simulink model "controller_observer_AllAgents_v5"  (Simulink 8.4) (continued)
== system controller_observer_AllAgents_v5/Controller == (continued)
  CornerClass1Pod2_Cooling_Mux/1 -> CornerClass1Pod2_Cooling/1(In1)
  [On_Off] -> CornerClass2Pod1_Cooling/Enable
  [On_Off] -> CornerClass1Pod2_Cooling/Enable
  [On_Off] -> CornerClass2Pod2_Cooling/Enable
  [On_Off] -> CornerClass1Pod3_Cooling/Enable
  [On_Off] -> CornerClass2Pod3_Cooling/Enable
  [On_Off] -> MultiClass1Pod1_Cooling/Enable
  [On_Off] -> MultiClass2Pod1_Cooling/Enable
  [On_Off] -> MultiClass1Pod2_Cooling/Enable
  [On_Off] -> MultiClass2Pod2_Cooling/Enable
  [On_Off] -> MultiClass1Pod3_Cooling/Enable
  [On_Off] -> MultiClass2Pod3_Cooling/Enable
  [On_Off] -> Mech_Cooling/Enable
  [On_Off] -> MainCorridor_Cooling/Enable
  [On_Off] -> CorridorPod3_Cooling/Enable
  [On_Off] -> CorridorPod2_Cooling/Enable
  [On_Off] -> CorridorPod1_Cooling/Enable
  [On_Off] -> ComputerClass_Cooling/Enable
  [On_Off] -> LibraryMediaCenter_Cooling/Enable
  [On_Off] -> Offices_Cooling/Enable
  [On_Off] -> Lobby_Cooling/Enable
  [On_Off] -> Bath_Cooling/Enable
  [On_Off] -> Gym_Cooling/Enable
  [On_Off] -> Kitchen_Cooling/Enable
  [On_Off] -> Cafeteria_Cooling/Enable
  [On_Off] -> CornerClass2Pod1_Heating/Enable
  [On_Off] -> CornerClass1Pod2_Heating/Enable
  [On_Off] -> CornerClass2Pod2_Heating/Enable
  [On_Off] -> CornerClass1Pod3_Heating/Enable
  [On_Off] -> CornerClass2Pod3_Heating/Enable
  [On_Off] -> MultiClass2Pod3_Heating/Enable
  [On_Off] -> MultiClass1Pod3_Heating/Enable
  [On_Off] -> MultiClass2Pod2_Heating/Enable
  [On_Off] -> MultiClass1Pod2_Heating/Enable
  [On_Off] -> MultiClass2Pod1_Heating/Enable
  [On_Off] -> MultiClass1Pod1_Heating/Enable
  [On_Off] -> CorridorPod1_Heating/Enable
  [On_Off] -> CorridorPod2_Heating/Enable
  [On_Off] -> CorridorPod3_Heating/Enable
  [On_Off] -> MainCorridor_Heating/Enable
  [On_Off] -> Mech_Heating/Enable
  [On_Off] -> Lobby_Heating/Enable
  [On_Off] -> Offices_Heating/Enable
  [On_Off] -> LibraryMediaCenter_Heating/Enable
  [On_Off] -> ComputerClass_Heating/Enable
  [On_Off] -> Cafeteria_Heating/Enable
  [On_Off] -> Kitchen_Heating/Enable
  [On_Off] -> Gym_Heating/Enable
  [On_Off] -> Bath_Heating/Enable
  Fault_Tz_CornerClass1Pod1/1(Out) -> [TzCornerClass1Pod1]
  Fault_Tsa_CornerClass1Pod1/1(Out) -> [TsaCornerClass1Pod1]
  Fault_Tc_CornerClass1Pod1_Cooling/1(Out) -> [TcCornerClass1Pod1_Cooling]
  Fault_Tc_CornerClass1Pod1_Heating/1(Out) -> [TcCornerClass1Pod1_Heating]
  Fault_Tz_CornerClass2Pod1/1(Out) -> [TzCornerClass2Pod1]
  Fault_Tsa_CornerClass2Pod1/1(Out) -> [TsaCornerClass2Pod1]
  Fault_Tc_CornerClass2Pod1_Cooling/1(Out) -> [TcCornerClass2Pod1_Cooling]
  Fault_Tc_CornerClass2Pod1_Heating/1(Out) -> [TcCornerClass2Pod1_Heating]
  Fault_Tz_CornerClass1Pod2/1(Out) -> [TzCornerClass1Pod2]
  Fault_Tsa_CornerClass1Pod2/1(Out) -> [TsaCornerClass1Pod2]
  Fault_Tc_CornerClass1Pod2_Cooling/1(Out) -> [TcCornerClass1Pod2_Cooling]
  Fault_Tc_CornerClass1Pod2_Heating/1(Out) -> [TcCornerClass1Pod2_Heating]
  Fault_Tz_CornerClass2Pod2/1(Out) -> [TzCornerClass2Pod2]
  Fault_Tsa_CornerClass2Pod2/1(Out) -> [TsaCornerClass2Pod2]
  Fault_Tc_CornerClass2Pod2_Cooling/1(Out) -> [TcCornerClass2Pod2_Cooling]
  Fault_Tc_CornerClass2Pod2_Heating/1(Out) -> [TcCornerClass2Pod2_Heating]
  Fault_Tz_CornerClass1Pod3/1(Out) -> [TzCornerClass1Pod3]
  Fault_Tsa_CornerClass1Pod3/1(Out) -> [TsaCornerClass1Pod3]
  Fault_Tc_CornerClass1Pod3_Cooling/1(Out) -> [TcCornerClass1Pod3_Cooling]
  Fault_Tc_CornerClass1Pod3_Heating/1(Out) -> [TcCornerClass1Pod3_Heating]
  Fault_Tz_CornerClass2Pod3/1(Out) -> [TzCornerClass2Pod3]
  Fault_Tsa_CornerClass2Pod3/1(Out) -> [TsaCornerClass2Pod3]
  Fault_Tc_CornerClass2Pod3_Cooling/1(Out) -> [TcCornerClass2Pod3_Cooling]
  Fault_Tc_CornerClass2Pod3_Heating/1(Out) -> [TcCornerClass2Pod3_Heating]
  Fault_Tz_MultiClass1Pod1/1(Out) -> [TzMultiClass1Pod1]
  Fault_Tsa_MultiClass1Pod1/1(Out) -> [TsaMultiClass1Pod1]
  Fault_Tc_MultiClass1Pod1_Cooling/1(Out) -> [TcMultiClass1Pod1_Cooling]
  Fault_Tc_MultiClass1Pod1_Heating/1(Out) -> [TcMultiClass1Pod1_Heating]
  Fault_Tz_MultiClass2Pod1/1(Out) -> [TzMultiClass2Pod1]
  Fault_Tsa_MultiClass2Pod1/1(Out) -> [TsaMultiClass2Pod1]
  Fault_Tc_MultiClass2Pod1_Cooling/1(Out) -> [TcMultiClass2Pod1_Cooling]
  Fault_Tc_MultiClass2Pod1_Heating/1(Out) -> [TcMultiClass2Pod1_Heating]
  Fault_Tz_MultiClass1Pod2/1(Out) -> [TzMultiClass1Pod2]
  Fault_Tsa_MultiClass1Pod2/1(Out) -> [TsaMultiClass1Pod2]
  Fault_Tc_MultiClass1Pod2_Cooling/1(Out) -> [TcMultiClass1Pod2_Cooling]
  Fault_Tc_MultiClass1Pod2_Heating/1(Out) -> [TcMultiClass1Pod2_Heating]
  Fault_Tc_MultiClass2Pod2_Cooling/1(Out) -> [TcMultiClass2Pod2_Cooling]
  Fault_Tsa_MultiClass2Pod2/1(Out) -> [TsaMultiClass2Pod2]
  Fault_Tz_MultiClass2Pod2/1(Out) -> [TzMultiClass2Pod2]
  Fault_Tc_MultiClass2Pod2_Heating/1(Out) -> [TcMultiClass2Pod2_Heating]
  Fault_Tz_MultiClass1Pod3/1(Out) -> [TzMultiClass1Pod3]
  Fault_Tc_MultiClass1Pod3_Cooling/1(Out) -> [TcMultiClass1Pod3_Cooling]
  Fault_Tsa_MultiClass1Pod3/1(Out) -> [TsaMultiClass1Pod3]
  Fault_Tc_MultiClass1Pod3_Heating/1(Out) -> [TcMultiClass1Pod3_Heating]
  Fault_Tz_MultiClass2Pod3/1(Out) -> [TzMultiClass2Pod3]
  Fault_Tc_MultiClass2Pod3_Cooling/1(Out) -> [TcMultiClass2Pod3_Cooling]
  Fault_Tsa_MultiClass2Pod3/1(Out) -> [TsaMultiClass2Pod3]
  Fault_Tc_MultiClass2Pod3_Heating/1(Out) -> [TcMultiClass2Pod3_Heating]
  Fault_Tz_CorridorPod1/1(Out) -> [TzCorridorPod1]
  Fault_Tsa_CorridorPod1/1(Out) -> [TsaCorridorPod1]
  Fault_Tc_CorridorPod1_Heating/1(Out) -> [TcCorridorPod1_Heating]
  Fault_Tc_CorridorPod1_Cooling/1(Out) -> [TcCorridorPod1_Cooling]
  Fault_Tz_CorridorPod2/1(Out) -> [TzCorridorPod2]
  Fault_Tc_CorridorPod2_Heating/1(Out) -> [TcCorridorPod2_Heating]
  Fault_Tc_CorridorPod2_Cooling/1(Out) -> [TcCorridorPod2_Cooling]
  Fault_Tsa_CorridorPod2/1(Out) -> [TsaCorridorPod2]
  Fault_Tz_CorridorPod3/1(Out) -> [TzCorridorPod3]
  Fault_Tc_CorridorPod3_Cooling/1(Out) -> [TcCorridorPod3_Cooling]
  Fault_Tsa_CorridorPod3/1(Out) -> [TsaCorridorPod3]
  Fault_Tc_CorridorPod3_Heating/1(Out) -> [TcCorridorPod3_Heating]
  Fault_Tc_MainCorridor_Heating/1(Out) -> [TcMainCorridor_Heating]
  Fault_Tc_MainCorridor_Cooling/1(Out) -> [TcMainCorridor_Cooling]
  Fault_Tz_MainCorridor/1(Out) -> [TzMainCorridor]
  Fault_Tsa_MainCorridor/1(Out) -> [TsaMainCorridor]
  Fault_Tz_Mech/1(Out) -> [TzMech]
  Fault_Tc_Mech_Cooling/1(Out) -> [TcMech_Cooling]
  Fault_Tc_Mech_Heating/1(Out) -> [TcMech_Heating]
  Fault_Tsa_Mech/1(Out) -> [TsaMech]
  Fault_Tc_ComputerClass_Heating/1(Out) -> [TcComputerClass_Heating]
  Fault_Tsa_ComputerClass/1(Out) -> [TsaComputerClass]
  Fault_Tz_ComputerClass/1(Out) -> [TzComputerClass]
  Fault_Tc_ComputerClass_Cooling/1(Out) -> [TcComputerClass_Cooling]
  Fault_Tc_LibraryMediaCenter_Cooling/1(Out) -> [TcLibrary_Cooling]
  Fault_Tsa_LibraryMediaCenter/1(Out) -> [TsaLibrary]
  Fault_Tz_LibraryMediaCenter/1(Out) -> [TzLibrary]
  Fault_Tc_LibraryMediaCenter_Heating/1(Out) -> [TcLibrary_Heating]
  Fault_Tc_Offices_Heating/1(Out) -> [TcOffices_Heating]
  Fault_Tz_Offices/1(Out) -> [TzOffices]
  Fault_Tsa_Offices/1(Out) -> [TsaOffices]
  Fault_Tc_Offices_Cooling/1(Out) -> [TcOffices_Cooling]
  Fault_Tc_Lobby_Cooling/1(Out) -> [TcLobby_Cooling]
  Fault_Tc_Lobby_Heating/1(Out) -> [TcLobby_Heating]
  Fault_Tsa_Lobby/1(Out) -> [TsaLobby]
  Fault_Tz_Lobby/1(Out) -> [TzLobby]
  Fault_Tc_Cafeteria_Cooling/1(Out) -> [TcCafeteria_Cooling]
  Fault_Tc_Cafeteria_Heating/1(Out) -> [TcCafeteria_Heating]
  Fault_Tz_Cafeteria/1(Out) -> [TzCafeteria]
  Fault_Tsa_Cafeteria/1(Out) -> [TsaCafeteria]
  Fault_Tc_Kitchen_Cooling/1(Out) -> [TcKitchen_Cooling]
  Fault_Tc_Kitchen_Heating/1(Out) -> [TcKitchen_Heating]
  Fault_Tsa_Kitchen/1(Out) -> [TsaKitchen]
  Fault_Tz_Kitchen/1(Out) -> [TzKitchen]
  Fault_Tsa_Gym/1(Out) -> [TsaGym]
  Fault_Tc_Gym_Cooling/1(Out) -> [TcGym_Cooling]
  Fault_Tz_Gym/1(Out) -> [TzGym]
  Fault_Tc_Gym_Heating/1(Out) -> [TcGym_Heating]
  Fault_Tz_Bath/1(Out) -> [TzBath]
  Fault_Tsa_Bath/1(Out) -> [TsaBath]
  Fault_Tc_Bath_Cooling/1(Out) -> [TcBath_Cooling]
  Fault_Tc_Bath_Heating/1(Out) -> [TcBath_Heating]
  Fault_Tst_Cooling/1(Out) -> [Tst_Cooling]
  Fault_Tamb/1(Out) -> [Tamb]
  Fault_Tst_Heating/1(Out) -> [Tst_Heating]
  Demux/104 -> [On_Off]
  Fault_mc_CornerClass1Pod1_Cooling/1(Out) -> Mux2/1
  Fault_mc_CornerClass2Pod1_Cooling/1(Out) -> Mux2/2
  Fault_mc_CornerClass1Pod2_Cooling/1(Out) -> Mux2/3
  Fault_mc_CornerClass2Pod2_Cooling/1(Out) -> Mux2/4
  Fault_mc_CornerClass1Pod3_Cooling/1(Out) -> Mux2/5
  Fault_mc_CornerClass2Pod3_Cooling/1(Out) -> Mux2/6
  Fault_mc_MultiClass1Pod2_Cooling/1(Out) -> Mux2/9
  Fault_mc_MultiClass1Pod1_Cooling/1(Out) -> Mux2/7
  Fault_mc_MultiClass1Pod3_Cooling/1(Out) -> Mux2/11
  Fault_mc_MultiClass2Pod1_Cooling/1(Out) -> Mux2/8
  Fault_mc_MultiClass2Pod3_Cooling/1(Out) -> Mux2/12
  Fault_mc_MultiClass2Pod2_Cooling/1(Out) -> Mux2/10
  Fault_mc_CorridorPod1_Cooling/1(Out) -> Mux2/13
  Fault_mc_CorridorPod3_Cooling/1(Out) -> Mux2/15
  Fault_mc_Mech_Cooling/1(Out) -> Mux2/17
  Fault_mc_CorridorPod2_Cooling/1(Out) -> Mux2/14
  Fault_mc_ComputerClass_Cooling/1(Out) -> Mux2/18
  Fault_mc_MainCorridor_Cooling/1(Out) -> Mux2/16
  Fault_mc_Gym_Cooling/1(Out) -> Mux2/24
  Fault_mc_Kitchen_Cooling/1(Out) -> Mux2/23
  Fault_mc_Lobby_Cooling/1(Out) -> Mux2/21
  Fault_mc_Cafeteria_Cooling/1(Out) -> Mux2/22
  Fault_mc_LibraryMediaCenter_Cooling/1(Out) -> Mux2/19
  Fault_mc_Offices_Cooling/1(Out) -> Mux2/20
  Fault_mc_Bath_Cooling/1(Out) -> Mux2/25
  Fault_mc_Bath_Heating/1(Out) -> Mux2/50
  Fault_mc_ComputerClass_Heating/1(Out) -> Mux2/43
  Fault_mc_CornerClass2Pod2_Heating/1(Out) -> Mux2/29
  Fault_mc_CorridorPod1_Heating/1(Out) -> Mux2/38
  Fault_mc_CornerClass1Pod3_Heating/1(Out) -> Mux2/30
  Fault_mc_CornerClass2Pod1_Heating/1(Out) -> Mux2/27
  Fault_mc_CornerClass1Pod1_Heating/1(Out) -> Mux2/26
  Fault_mc_CornerClass1Pod2_Heating/1(Out) -> Mux2/28
  Fault_mc_Cafeteria_Heating/1(Out) -> Mux2/47
  Fault_mc_CornerClass2Pod3_Heating/1(Out) -> Mux2/31
  Fault_mc_MainCorridor_Heating/1(Out) -> Mux2/41
  Fault_mc_Kitchen_Heating/1(Out) -> Mux2/48
  Fault_mc_Gym_Heating/1(Out) -> Mux2/49
  Fault_mc_Lobby_Heating/1(Out) -> Mux2/46
  Fault_mc_CorridorPod3_Heating/1(Out) -> Mux2/40
  Fault_mc_CorridorPod2_Heating/1(Out) -> Mux2/39
  Fault_mc_LibraryMediaCenter_Heating/1(Out) -> Mux2/44
  Fault_mc_Mech_Heating/1(Out) -> Mux2/42
  Fault_mc_MultiClass1Pod1_Heating/1(Out) -> Mux2/32
  Fault_mc_MultiClass2Pod2_Heating/1(Out) -> Mux2/35
  Fault_mc_MultiClass1Pod3_Heating/1(Out) -> Mux2/36
  Fault_mc_MultiClass2Pod3_Heating/1(Out) -> Mux2/37
  Fault_mc_MultiClass1Pod2_Heating/1(Out) -> Mux2/34
  Fault_mc_MultiClass2Pod1_Heating/1(Out) -> Mux2/33
  Fault_mc_Offices_Heating/1(Out) -> Mux2/45
  CorridorPod2_Mux1/1 -> CorridorPod2_Agent/1(In1)
  [Tamb] -> MainCorridor_Mux1/2
  [TcLibrary_Cooling] -> LibraryMediaCenter_Mux1/3
  LibraryMediaCenter_Mux1/1 -> LibraryMediaCenter_Agent/1(In1)
  [TzMainCorridor] -> MainCorridor_Mux1/1
  MainCorridor_Mux1/1 -> MainCorridor_Agent/1(In1)
  [Tamb] -> LibraryMediaCenter_Mux1/2
  [Tamb] -> CorridorPod3_Mux1/2
  [TzMultiClass2Pod3] -> MultiClass2Pod3_Mux1/1
  MultiClass1Pod2_Mux1/1 -> MultiClass1Pod2_Agent/1(In1)
  [Tamb] -> MultiClass1Pod2_Mux1/2
  [TzMultiClass2Pod1] -> MultiClass2Pod1_Mux1/1
  [Tamb] -> MultiClass2Pod1_Mux1/2
  [TcCornerClass2Pod3_Cooling] -> CornerClass2Pod3_Mux1/3
  [Tamb] -> MultiClass1Pod1_Mux1/2
  [TcCornerClass1Pod3_Cooling] -> CornerClass1Pod3_Mux1/3
  [Tamb] -> CornerClass2Pod2_Mux1/2
  [Tamb] -> CornerClass1Pod2_Mux1/2
  Cafeteria_Mux1/1 -> Cafeteria_Agent/1(In1)
  [Tamb] -> MultiClass2Pod2_Mux1/2
  CornerClass1Pod3_Mux1/1 -> CornerClass1Pod3_Agent/1(In1)
  [Tamb] -> MultiClass2Pod3_Mux1/2
  [Tamb] -> Bath_Mux1/2
  [TcMultiClass1Pod1_Cooling] -> MultiClass1Pod1_Mux1/3
  Lobby_Mux1/1 -> Lobby_Agent/1(In1)
  [Tamb] -> Lobby_Mux1/2
  [TcCafeteria_Cooling] -> Cafeteria_Mux1/3
  Offices_Mux1/1 -> Offices_Agent/1(In1)
  [TcMech_Cooling] -> Mech_Mux1/3
  [Tamb] -> Cafeteria_Mux1/2
  [TzMultiClass1Pod1] -> MultiClass1Pod1_Mux1/1
  [TcComputerClass_Cooling] -> ComputerClass_Mux1/3
  [Tamb] -> CornerClass1Pod3_Mux1/2
  [Tamb] -> CorridorPod1_Mux1/2
  [TzOffices] -> Offices_Mux1/1
  [Tamb] -> Gym_Mux1/2
  [TzMech] -> Mech_Mux1/1
  ComputerClass_Mux1/1 -> ComputerClass_Agent/1(In1)
  [TzCorridorPod1] -> CorridorPod1_Mux1/1
  [Tamb] -> ComputerClass_Mux1/2
  MultiClass1Pod1_Mux1/1 -> MultiClass1Pod1_Agent/1(In1)
  Bath_Mux1/1 -> Bath_Agent/1(In1)
  MultiClass2Pod3_Mux1/1 -> MultiClass2Pod3_Agent/1(In1)
  [TzLibrary] -> LibraryMediaCenter_Mux1/1
  [TzLobby] -> Lobby_Mux1/1
  [Tst_Cooling] -> CornerClass1Pod1_Mux1/6
  [Tamb] -> MultiClass1Pod3_Mux1/2
  [TzCornerClass1Pod2] -> CornerClass1Pod2_Mux1/1
  [TzCorridorPod3] -> CorridorPod3_Mux1/1
  [TcCorridorPod2_Cooling] -> CorridorPod2_Mux1/3
  MultiClass2Pod2_Mux1/1 -> MultiClass2Pod2_Agent/1(In1)
  CornerClass1Pod1_Mux1/1 -> CornerClass1Pod1_Agent/1(In1)
  [TzNeighborCornerClass1Pod1] -> CornerClass1Pod1_Mux1/8
  [TcLobby_Cooling] -> Lobby_Mux1/3
  [TzGym] -> Gym_Mux1/1
  [TcMultiClass2Pod2_Cooling] -> MultiClass2Pod2_Mux1/3
  [TzCornerClass1Pod3] -> CornerClass1Pod3_Mux1/1
  MultiClass2Pod1_Mux1/1 -> MultiClass2Pod1_Agent/1(In1)
  [TcGym_Cooling] -> Gym_Mux1/3
  [TzMultiClass1Pod3] -> MultiClass1Pod3_Mux1/1
  [TcCornerClass2Pod2_Cooling] -> CornerClass2Pod2_Mux1/3
  [Tamb] -> CornerClass1Pod1_Mux1/2
  CorridorPod1_Mux1/1 -> CorridorPod1_Agent/1(In1)
  [TzMultiClass1Pod2] -> MultiClass1Pod2_Mux1/1
  Kitchen_Mux1/1 -> Kitchen_Agent/1(In1)
  [Tamb] -> CornerClass2Pod1_Mux1/2
  Gym_Mux1/1 -> Gym_Agent/1(In1)
  [TsaCornerClass1Pod1] -> CornerClass1Pod1_Mux1/5
  [TzCornerClass2Pod3] -> CornerClass2Pod3_Mux1/1
  [TcCornerClass1Pod1_Cooling] -> CornerClass1Pod1_Mux1/3
  CornerClass2Pod3_Mux1/1 -> CornerClass2Pod3_Agent/1(In1)
  [TcKitchen_Cooling] -> Kitchen_Mux1/3
  [TzCornerClass2Pod1] -> CornerClass2Pod1_Mux1/1
  [Tamb] -> CorridorPod2_Mux1/2
  [TcMultiClass1Pod3_Cooling] -> MultiClass1Pod3_Mux1/3
  CornerClass2Pod2_Mux1/1 -> CornerClass2Pod2_Agent/1(In1)
  CornerClass1Pod2_Mux1/1 -> CornerClass1Pod2_Agent/1(In1)
  [Tamb] -> Mech_Mux1/2
  [Tamb] -> Kitchen_Mux1/2
  [TcMultiClass2Pod1_Cooling] -> MultiClass2Pod1_Mux1/3
  CorridorPod3_Mux1/1 -> CorridorPod3_Agent/1(In1)
  [Tamb] -> Offices_Mux1/2
  [Tamb] -> CornerClass2Pod3_Mux1/2
  [TzMultiClass2Pod2] -> MultiClass2Pod2_Mux1/1
  [TzKitchen] -> Kitchen_Mux1/1
  [TzCafeteria] -> Cafeteria_Mux1/1
  [TcOffices_Cooling] -> Offices_Mux1/3
  [TcCorridorPod1_Cooling] -> CorridorPod1_Mux1/3
  [TcMultiClass1Pod2_Cooling] -> MultiClass1Pod2_Mux1/3
  [TcCornerClass2Pod1_Cooling] -> CornerClass2Pod1_Mux1/3
  [TcMultiClass2Pod3_Cooling] -> MultiClass2Pod3_Mux1/3
  [TcCornerClass1Pod2_Cooling] -> CornerClass1Pod2_Mux1/3
  [TzBath] -> Bath_Mux1/1
  MultiClass1Pod3_Mux1/1 -> MultiClass1Pod3_Agent/1(In1)
  Mech_Mux1/1 -> Mech_Agent/1(In1)
  [TzCorridorPod2] -> CorridorPod2_Mux1/1
  [TzCornerClass2Pod2] -> CornerClass2Pod2_Mux1/1
  [TzCornerClass1Pod1] -> CornerClass1Pod1_Mux1/1
  [TcBath_Cooling] -> Bath_Mux1/3
  CornerClass2Pod1_Mux1/1 -> CornerClass2Pod1_Agent/1(In1)
  [TcCorridorPod3_Cooling] -> CorridorPod3_Mux1/3
  [TzComputerClass] -> ComputerClass_Mux1/1
  [TcMainCorridor_Cooling] -> MainCorridor_Mux1/3
  [On_Off] -> CornerClass2Pod1_Agent/Enable
  [On_Off] -> CornerClass1Pod2_Agent/Enable
  [On_Off] -> CornerClass2Pod2_Agent/Enable
  [On_Off] -> CornerClass1Pod3_Agent/Enable
  [On_Off] -> CornerClass2Pod3_Agent/Enable
  [On_Off] -> MultiClass1Pod1_Agent/Enable
  [On_Off] -> MultiClass2Pod1_Agent/Enable
  [On_Off] -> MultiClass1Pod2_Agent/Enable
  [On_Off] -> MultiClass2Pod2_Agent/Enable
  [On_Off] -> MultiClass1Pod3_Agent/Enable
  [On_Off] -> MultiClass2Pod3_Agent/Enable
  [On_Off] -> CorridorPod1_Agent/Enable
  [On_Off] -> CorridorPod2_Agent/Enable
  [On_Off] -> CorridorPod3_Agent/Enable
  [On_Off] -> MainCorridor_Agent/Enable
  [On_Off] -> Mech_Agent/Enable
  [On_Off] -> ComputerClass_Agent/Enable
  [On_Off] -> LibraryMediaCenter_Agent/Enable
  [On_Off] -> Offices_Agent/Enable
  [On_Off] -> Lobby_Agent/Enable
  [On_Off] -> Cafeteria_Agent/Enable
  [On_Off] -> Kitchen_Agent/Enable
  [On_Off] -> Gym_Agent/Enable
  [On_Off] -> Bath_Agent/Enable
  Mux3/1 -> [DzNeighborCornerClass1Pod1]
  [DzMultiClass1Pod1] -> Mux3/1
  [DzCorridorPod1] -> Mux3/2
  Mux4/1 -> [DzNeighborCornerClass2Pod1]
  Mux5/1 -> [DzNeighborCornerClass1Pod2]
  [DzMultiClass1Pod2] -> Mux5/1
  [DzCorridorPod2] -> Mux5/2
  Mux6/1 -> [DzNeighborCornerClass2Pod2]
  Mux7/1 -> [DzNeighborCornerClass1Pod3]
  [DzMultiClass1Pod3] -> Mux7/1
  [DzCorridorPod3] -> Mux7/2
  Mux8/1 -> [DzNeighborCornerClass2Pod3]
  [DzCorridorPod1] -> Mux4/1
  [DzMultiClass2Pod1] -> Mux4/2
  [DzCorridorPod2] -> Mux6/1
  [DzMultiClass2Pod2] -> Mux6/2
  [DzCorridorPod3] -> Mux8/1
  [DzMultiClass2Pod3] -> Mux8/2
  Mux9/1 -> [DzNeighborMultiClass1Pod1]
  Mux10/1 -> [DzNeighborMultiClass2Pod1]
  Mux11/1 -> [DzNeighborMultiClass1Pod2]
  Mux12/1 -> [DzNeighborMultiClass2Pod2]
  Mux13/1 -> [DzNeighborMultiClass1Pod3]
  [DzCorridorPod3] -> Mux13/2
  Mux14/1 -> [DzNeighborMultiClass2Pod3]
  [DzCornerClass1Pod1] -> Mux9/3
  [DzCorridorPod1] -> Mux9/2
  [DzLobby] -> Mux9/1
  [DzCorridorPod1] -> Mux10/1
  [DzMainCorridor] -> Mux10/2
  [DzCornerClass2Pod1] -> Mux10/3
  [DzCorridorPod2] -> Mux11/2
  [DzCornerClass1Pod2] -> Mux11/3
  [DzMainCorridor] -> Mux11/1
  [DzCorridorPod2] -> Mux12/1
  [DzMainCorridor] -> Mux12/2
  [DzCornerClass2Pod2] -> Mux12/3
  [DzLibrary] -> Mux13/1
  [DzCornerClass1Pod3] -> Mux13/3
  [DzCorridorPod3] -> Mux14/1
  [DzComputerClass] -> Mux14/2
  [DzCornerClass2Pod3] -> Mux14/3
  Mux15/1 -> [DzNeighborCorridorPod1]
  [DzCornerClass1Pod1] -> Mux15/1
  [DzMultiClass2Pod1] -> Mux15/4
  Mux16/1 -> [DzNeighborCorridorPod2]
  [DzCornerClass1Pod2] -> Mux16/1
  [DzMultiClass2Pod2] -> Mux16/4
  Mux17/1 -> [DzNeighborCorridorPod3]
  [DzCornerClass1Pod3] -> Mux17/1
  Mux18/1 -> [DzNeighborMainCorridor]
  Mux19/1 -> [DzNeighborMech]
  [DzMainCorridor] -> Mux15/3
  [DzCornerClass2Pod1] -> Mux15/5
  [DzMultiClass1Pod1] -> Mux15/2
  [DzMultiClass1Pod2] -> Mux16/2
  [DzMainCorridor] -> Mux16/3
  [DzCornerClass2Pod2] -> Mux16/5
  [DzMultiClass1Pod3] -> Mux17/2
  [DzLibrary] -> Mux17/3
  [DzComputerClass] -> Mux17/4
  [DzMultiClass2Pod3] -> Mux17/5
  [DzCornerClass2Pod3] -> Mux17/6
  [DzBath] -> Mux18/3
  [DzMech] -> Mux18/2
  [DzLobby] -> Mux18/1
  [DzMultiClass2Pod2] -> Mux18/4
  [DzCorridorPod2] -> Mux18/5
  [DzMultiClass1Pod2] -> Mux18/6
  [DzCorridorPod1] -> Mux18/8
  [DzMultiClass2Pod1] -> Mux18/7
  [DzLobby] -> Mux19/1
  [DzOffices] -> Mux19/2
  [DzGym] -> Mux19/3
  [DzKitchen] -> Mux19/4
  [DzCafeteria] -> Mux19/5
  [DzMainCorridor] -> Mux19/7
  [DzBath] -> Mux19/6
  Mux20/1 -> [DzNeighborComputerClass]
  Mux21/1 -> [DzNeighborLibrary]
  [DzComputerClass] -> Mux21/2
  [DzCorridorPod3] -> Mux21/3
  Mux28/1 -> [DzNeighborOffices]
  Mux29/1 -> [DzNeighborLobby]
  [DzCorridorPod3] -> Mux20/1
  [DzLibrary] -> Mux20/2
  [DzMultiClass2Pod3] -> Mux20/3
  [DzMultiClass1Pod3] -> Mux21/4
  [DzBath] -> Mux21/1
  [DzLobby] -> Mux28/3
  [DzGym] -> Mux28/1
  [DzMech] -> Mux28/2
  [DzMainCorridor] -> Mux29/3
  [DzMultiClass1Pod1] -> Mux29/4
  [DzMech] -> Mux29/2
  [DzOffices] -> Mux29/1
  Mux30/1 -> [DzNeighborCafeteria]
  [DzKitchen] -> Mux30/1
  Mux31/1 -> [DzNeighborKitchen]
  [DzGym] -> Mux31/1
  Mux32/1 -> [DzNeighborGym]
  [DzOffices] -> Mux32/1
  Mux33/1 -> [DzNeighborBath]
  [DzMainCorridor] -> Mux33/1
  [DzMech] -> Mux33/2
  [DzLibrary] -> Mux33/4
  [DzCafeteria] -> Mux33/3
  [DzBath] -> Mux30/2
  [DzMech] -> Mux30/3
  [DzCafeteria] -> Mux31/2
  [DzMech] -> Mux31/3
  [DzKitchen] -> Mux32/2
  [DzMech] -> Mux32/3
  CornerClass1Pod1_Agent/1(Dz) -> [DzCornerClass1Pod1]
  CornerClass2Pod1_Agent/1(Dz) -> [DzCornerClass2Pod1]
  CornerClass1Pod2_Agent/1(Dz) -> [DzCornerClass1Pod2]
  CornerClass2Pod2_Agent/1(Dz) -> [DzCornerClass2Pod2]
  CornerClass1Pod3_Agent/1(Dz) -> [DzCornerClass1Pod3]
  CornerClass2Pod3_Agent/1(Dz) -> [DzCornerClass2Pod3]
  MultiClass1Pod1_Agent/1(Dz) -> [DzMultiClass1Pod1]
  MultiClass2Pod1_Agent/1(Dz) -> [DzMultiClass2Pod1]
  MultiClass1Pod3_Agent/1(Dz) -> [DzMultiClass1Pod3]
  MultiClass2Pod3_Agent/1(Dz) -> [DzMultiClass2Pod3]
  MultiClass1Pod2_Agent/1(Dz) -> [DzMultiClass1Pod2]
  MultiClass2Pod2_Agent/1(Dz) -> [DzMultiClass2Pod2]
  CorridorPod1_Agent/1(Dz) -> [DzCorridorPod1]
  CorridorPod2_Agent/1(Dz) -> [DzCorridorPod2]
  CorridorPod3_Agent/1(Dz) -> [DzCorridorPod3]
  MainCorridor_Agent/1(Dz) -> [DzMainCorridor]
  Mech_Agent/1(Dz) -> [DzMech]
  ComputerClass_Agent/1(Dz) -> [DzComputerClass]
  LibraryMediaCenter_Agent/1(Dz) -> [DzLibrary]
  Offices_Agent/1(Dz) -> [DzOffices]
  Lobby_Agent/1(Dz) -> [DzLobby]
  Cafeteria_Agent/1(Dz) -> [DzCafeteria]
  Kitchen_Agent/1(Dz) -> [DzKitchen]
  Gym_Agent/1(Dz) -> [DzGym]
  Bath_Agent/1(Dz) -> [DzBath]
  [TcCornerClass1Pod1_Heating] -> CornerClass1Pod1_Mux1/4
  [Tst_Heating] -> CornerClass1Pod1_Mux1/7
  [mcCornerClass1Pod1_Heating] -> CornerClass1Pod1_Mux1/10
  [mcCornerClass2Pod1_Cooling] -> CornerClass2Pod1_Mux1/9
  [TzNeighborCornerClass2Pod1] -> CornerClass2Pod1_Mux1/8
  [Tst_Heating] -> CornerClass2Pod1_Mux1/7
  [Tst_Cooling] -> CornerClass2Pod1_Mux1/6
  [TsaCornerClass2Pod1] -> CornerClass2Pod1_Mux1/5
  [TcCornerClass2Pod1_Heating] -> CornerClass2Pod1_Mux1/4
  [mcCornerClass2Pod1_Heating] -> CornerClass2Pod1_Mux1/10
  [mcCornerClass1Pod2_Cooling] -> CornerClass1Pod2_Mux1/9
  [TzNeighborCornerClass1Pod2] -> CornerClass1Pod2_Mux1/8
  [Tst_Cooling] -> CornerClass1Pod2_Mux1/6
  [TsaCornerClass1Pod2] -> CornerClass1Pod2_Mux1/5
  [TcCornerClass1Pod2_Heating] -> CornerClass1Pod2_Mux1/4
  [Tst_Heating] -> CornerClass1Pod2_Mux1/7
  [mcCornerClass1Pod2_Heating] -> CornerClass1Pod2_Mux1/10
  [mcCornerClass2Pod2_Cooling] -> CornerClass2Pod2_Mux1/9
  [TzNeighborCornerClass2Pod2] -> CornerClass2Pod2_Mux1/8
  [Tst_Cooling] -> CornerClass2Pod2_Mux1/6
  [TsaCornerClass2Pod2] -> CornerClass2Pod2_Mux1/5
  [TcCornerClass2Pod2_Heating] -> CornerClass2Pod2_Mux1/4
  [Tst_Heating] -> CornerClass2Pod2_Mux1/7
  [mcCornerClass2Pod2_Heating] -> CornerClass2Pod2_Mux1/10
  [mcCornerClass1Pod3_Cooling] -> CornerClass1Pod3_Mux1/9
  [TzNeighborCornerClass1Pod3] -> CornerClass1Pod3_Mux1/8
  [Tst_Cooling] -> CornerClass1Pod3_Mux1/6
  [TsaCornerClass1Pod3] -> CornerClass1Pod3_Mux1/5
  [TcCornerClass1Pod3_Heating] -> CornerClass1Pod3_Mux1/4
  [Tst_Heating] -> CornerClass1Pod3_Mux1/7
  [mcCornerClass1Pod3_Heating] -> CornerClass1Pod3_Mux1/10
  [mcCornerClass2Pod3_Cooling] -> CornerClass2Pod3_Mux1/9
  [TzNeighborCornerClass2Pod3] -> CornerClass2Pod3_Mux1/8
  [Tst_Cooling] -> CornerClass2Pod3_Mux1/6
  [TsaCornerClass2Pod3] -> CornerClass2Pod3_Mux1/5
  [TcCornerClass2Pod3_Heating] -> CornerClass2Pod3_Mux1/4
  [Tst_Heating] -> CornerClass2Pod3_Mux1/7
  [mcCornerClass2Pod3_Heating] -> CornerClass2Pod3_Mux1/10
  [mcMultiClass1Pod1_Cooling] -> MultiClass1Pod1_Mux1/9
  [TzNeighborMultiClass1Pod1] -> MultiClass1Pod1_Mux1/8
  [TcMultiClass1Pod1_Heating] -> MultiClass1Pod1_Mux1/4
  [TsaMultiClass1Pod1] -> MultiClass1Pod1_Mux1/5
  [Tst_Cooling] -> MultiClass1Pod1_Mux1/6
  [Tst_Heating] -> MultiClass1Pod1_Mux1/7
  [mcMultiClass1Pod1_Heating] -> MultiClass1Pod1_Mux1/10
  [mcMultiClass2Pod1_Cooling] -> MultiClass2Pod1_Mux1/9
  [TzNeighborMultiClass2Pod1] -> MultiClass2Pod1_Mux1/8
  [Tst_Cooling] -> MultiClass2Pod1_Mux1/6
  [TsaMultiClass2Pod1] -> MultiClass2Pod1_Mux1/5
  [TcMultiClass2Pod1_Heating] -> MultiClass2Pod1_Mux1/4
  [Tst_Heating] -> MultiClass2Pod1_Mux1/7
  [mcMultiClass2Pod1_Heating] -> MultiClass2Pod1_Mux1/10
  [mcMultiClass1Pod2_Cooling] -> MultiClass1Pod2_Mux1/9
  [TzNeighborMultiClass1Pod2] -> MultiClass1Pod2_Mux1/8
  [Tst_Cooling] -> MultiClass1Pod2_Mux1/6
  [TsaMultiClass1Pod2] -> MultiClass1Pod2_Mux1/5
  [TcMultiClass1Pod2_Heating] -> MultiClass1Pod2_Mux1/4
  [Tst_Heating] -> MultiClass1Pod2_Mux1/7
  [mcMultiClass1Pod2_Heating] -> MultiClass1Pod2_Mux1/10
  [mcMultiClass2Pod2_Cooling] -> MultiClass2Pod2_Mux1/9
  [TzNeighborMultiClass2Pod2] -> MultiClass2Pod2_Mux1/8
  [Tst_Cooling] -> MultiClass2Pod2_Mux1/6
  [TsaMultiClass2Pod2] -> MultiClass2Pod2_Mux1/5
  [mcMultiClass2Pod2_Heating] -> MultiClass2Pod2_Mux1/10
  [Tst_Heating] -> MultiClass2Pod2_Mux1/7
  [TcMultiClass2Pod2_Heating] -> MultiClass2Pod2_Mux1/4
  [mcMultiClass1Pod3_Cooling] -> MultiClass1Pod3_Mux1/9
  [TzNeighborMultiClass1Pod3] -> MultiClass1Pod3_Mux1/8
  [Tst_Cooling] -> MultiClass1Pod3_Mux1/6
  [TsaMultiClass1Pod3] -> MultiClass1Pod3_Mux1/5
  [TcMultiClass1Pod3_Heating] -> MultiClass1Pod3_Mux1/4
  [Tst_Heating] -> MultiClass1Pod3_Mux1/7
  [mcMultiClass1Pod3_Heating] -> MultiClass1Pod3_Mux1/10
  [mcMultiClass2Pod3_Cooling] -> MultiClass2Pod3_Mux1/9
  [TzNeighborMultiClass2Pod3] -> MultiClass2Pod3_Mux1/8
  [Tst_Cooling] -> MultiClass2Pod3_Mux1/6
  [TsaMultiClass2Pod3] -> MultiClass2Pod3_Mux1/5
  [Tst_Heating] -> MultiClass2Pod3_Mux1/7
  [TcMultiClass2Pod3_Heating] -> MultiClass2Pod3_Mux1/4
  [mcMultiClass2Pod3_Heating] -> MultiClass2Pod3_Mux1/10
  [mcCorridorPod1_Cooling] -> CorridorPod1_Mux1/9
  [TzNeighborCorridorPod1] -> CorridorPod1_Mux1/8
  [Tst_Cooling] -> CorridorPod1_Mux1/6
  [TsaCorridorPod1] -> CorridorPod1_Mux1/5
  [TcCorridorPod1_Heating] -> CorridorPod1_Mux1/4
  [Tst_Heating] -> CorridorPod1_Mux1/7
  [mcCorridorPod1_Heating] -> CorridorPod1_Mux1/10
  [mcCorridorPod2_Cooling] -> CorridorPod2_Mux1/9
  [TzNeighborCorridorPod2] -> CorridorPod2_Mux1/8
  [Tst_Cooling] -> CorridorPod2_Mux1/6
  [Tst_Heating] -> CorridorPod2_Mux1/7
  [TsaCorridorPod2] -> CorridorPod2_Mux1/5
  [TcCorridorPod2_Heating] -> CorridorPod2_Mux1/4
  [mcCorridorPod2_Heating] -> CorridorPod2_Mux1/10
  [mcCorridorPod3_Cooling] -> CorridorPod3_Mux1/9
  [TzNeighborCorridorPod3] -> CorridorPod3_Mux1/8
  [Tst_Cooling] -> CorridorPod3_Mux1/6
  [TsaCorridorPod3] -> CorridorPod3_Mux1/5
  [TcCorridorPod3_Heating] -> CorridorPod3_Mux1/4
  [Tst_Heating] -> CorridorPod3_Mux1/7
  [mcCorridorPod3_Heating] -> CorridorPod3_Mux1/10
  [mcMainCorridor_Cooling] -> MainCorridor_Mux1/9
  [TzNeighborMainCorridor] -> MainCorridor_Mux1/8
  [Tst_Cooling] -> MainCorridor_Mux1/6
  [TsaMainCorridor] -> MainCorridor_Mux1/5
  [TcMainCorridor_Heating] -> MainCorridor_Mux1/4
  [Tst_Heating] -> MainCorridor_Mux1/7
  [mcMainCorridor_Heating] -> MainCorridor_Mux1/10
  [mcMech_Cooling] -> Mech_Mux1/9
  [TzNeighborMech] -> Mech_Mux1/8
  [Tst_Cooling] -> Mech_Mux1/6
  [TsaMech] -> Mech_Mux1/5
  [TcMech_Heating] -> Mech_Mux1/4
  [Tst_Heating] -> Mech_Mux1/7
  [mcMech_Heating] -> Mech_Mux1/10
  [TsaComputerClass] -> ComputerClass_Mux1/5
  [Tst_Cooling] -> ComputerClass_Mux1/6
  [TzNeighborComputerClass] -> ComputerClass_Mux1/8
  [mcComputerClass_Cooling] -> ComputerClass_Mux1/9
  [TcComputerClass_Heating] -> ComputerClass_Mux1/4
  [Tst_Heating] -> ComputerClass_Mux1/7
  [mcComputerClass_Heating] -> ComputerClass_Mux1/10
  [mcLibrary_Cooling] -> LibraryMediaCenter_Mux1/9
  [TzNeighborLibrary] -> LibraryMediaCenter_Mux1/8
  [Tst_Cooling] -> LibraryMediaCenter_Mux1/6
  [TsaLibrary] -> LibraryMediaCenter_Mux1/5
  [Tst_Heating] -> LibraryMediaCenter_Mux1/7
  [mcLibrary_Heating] -> LibraryMediaCenter_Mux1/10
  [TcLibrary_Heating] -> LibraryMediaCenter_Mux1/4
  [mcOffices_Cooling] -> Offices_Mux1/9
  [TzNeighborOffices] -> Offices_Mux1/8
  [Tst_Cooling] -> Offices_Mux1/6
  [TsaOffices] -> Offices_Mux1/5
  [TcOffices_Heating] -> Offices_Mux1/4
  [Tst_Heating] -> Offices_Mux1/7
  [mcOffices_Heating] -> Offices_Mux1/10
  [mcLobby_Cooling] -> Lobby_Mux1/9
  [TzNeighborLobby] -> Lobby_Mux1/8
  [Tst_Cooling] -> Lobby_Mux1/6
  [TsaLobby] -> Lobby_Mux1/5
  [TcLobby_Heating] -> Lobby_Mux1/4
  [Tst_Heating] -> Lobby_Mux1/7
  [mcLobby_Heating] -> Lobby_Mux1/10
  [mcCafeteria_Cooling] -> Cafeteria_Mux1/9
  [TzNeighborCafeteria] -> Cafeteria_Mux1/8
  [Tst_Cooling] -> Cafeteria_Mux1/6
  [TsaCafeteria] -> Cafeteria_Mux1/5
  [Tst_Heating] -> Cafeteria_Mux1/7
  [mcCafeteria_Heating] -> Cafeteria_Mux1/10
  [TcCafeteria_Heating] -> Cafeteria_Mux1/4
  [mcKitchen_Cooling] -> Kitchen_Mux1/9
  [TzNeighborKitchen] -> Kitchen_Mux1/8
  [Tst_Cooling] -> Kitchen_Mux1/6
  [TsaKitchen] -> Kitchen_Mux1/5
  [TcKitchen_Heating] -> Kitchen_Mux1/4
  [Tst_Heating] -> Kitchen_Mux1/7
  [mcKitchen_Heating] -> Kitchen_Mux1/10
  [mcGym_Cooling] -> Gym_Mux1/9
  [TzNeighborGym] -> Gym_Mux1/8
  [Tst_Cooling] -> Gym_Mux1/6
  [TsaGym] -> Gym_Mux1/5
  [TcGym_Heating] -> Gym_Mux1/4
  [Tst_Heating] -> Gym_Mux1/7
  [mcGym_Heating] -> Gym_Mux1/10
  [mcBath_Cooling] -> Bath_Mux1/9
  [TzNeighborBath] -> Bath_Mux1/8
  [Tst_Cooling] -> Bath_Mux1/6
  [TsaBath] -> Bath_Mux1/5
  [TcBath_Heating] -> Bath_Mux1/4
  [Tst_Heating] -> Bath_Mux1/7
  [mcBath_Heating] -> Bath_Mux1/10
  EnableDifonifmode1/1(enable detection) -> EnableD_H_CornerClass1Pod1_mat/1
  [mcCornerClass1Pod1_Cooling] -> CornerClass1Pod1_Mux1/9
  EnableDifonifmode2/1(enable detection) -> EnableD_C_CornerClass1Pod1_mat/1
  [On_Off] -> CornerClass1Pod1_Agent/Enable
  [On_Off] -> EnableDifonifmode2/2(on_off), EnableDifonifmode1/2(on_off)
  [mcCornerClass1Pod1_Cooling] -> EnableDifonifmode2/1(mc)
  [mcCornerClass1Pod1_Heating] -> EnableDifonifmode1/1(mc)
  EnableDifonifmode3/1(enable detection) -> EnableD_H_CornerClass2Pod1_mat/1
  EnableDifonifmode4/1(enable detection) -> EnableD_C_CornerClass2Pod1_mat/1
  [On_Off] -> EnableDifonifmode4/2(on_off), EnableDifonifmode3/2(on_off)
  [mcCornerClass2Pod1_Cooling] -> EnableDifonifmode4/1(mc)
  [mcCornerClass2Pod1_Heating] -> EnableDifonifmode3/1(mc)
  EnableDifonifmode5/1(enable detection) -> EnableD_H_CornerClass1Pod2_mat/1
  EnableDifonifmode6/1(enable detection) -> EnableD_C_CornerClass1Pod2_mat/1
  [On_Off] -> EnableDifonifmode6/2(on_off), EnableDifonifmode5/2(on_off)
  [mcCornerClass1Pod2_Cooling] -> EnableDifonifmode6/1(mc)
  [mcCornerClass1Pod2_Heating] -> EnableDifonifmode5/1(mc)
  EnableDifonifmode7/1(enable detection) -> EnableD_H_CornerClass2Pod2_mat/1
  EnableDifonifmode8/1(enable detection) -> EnableD_C_CornerClass2Pod2_mat/1
  [On_Off] -> EnableDifonifmode8/2(on_off), EnableDifonifmode7/2(on_off)
  [mcCornerClass2Pod2_Cooling] -> EnableDifonifmode8/1(mc)
  [mcCornerClass2Pod2_Heating] -> EnableDifonifmode7/1(mc)
  EnableDifonifmode9/1(enable detection) -> EnableD_H_CornerClass1Pod3_mat/1
  EnableDifonifmode10/1(enable detection) -> EnableD_C_CornerClass1Pod3_mat/1
  [On_Off] -> EnableDifonifmode10/2(on_off), EnableDifonifmode9/2(on_off)
  [mcCornerClass1Pod3_Cooling] -> EnableDifonifmode10/1(mc)
  [mcCornerClass1Pod3_Heating] -> EnableDifonifmode9/1(mc)
  EnableDifonifmode11/1(enable detection) -> EnableD_H_CornerClass2Pod3_mat/1
  EnableDifonifmode12/1(enable detection) -> EnableD_C_CornerClass2Pod3_mat/1
  [On_Off] -> EnableDifonifmode12/2(on_off), EnableDifonifmode11/2(on_off)
  [mcCornerClass2Pod3_Cooling] -> EnableDifonifmode12/1(mc)
  [mcCornerClass2Pod3_Heating] -> EnableDifonifmode11/1(mc)
  EnableDifonifmode13/1(enable detection) -> EnableD_H_MultiClass2Pod2_mat/1
  EnableDifonifmode14/1(enable detection) -> EnableD_C_MultiClass2Pod2_mat/1
  [On_Off] -> EnableDifonifmode14/2(on_off), EnableDifonifmode13/2(on_off)
  [mcMultiClass2Pod2_Cooling] -> EnableDifonifmode14/1(mc)
  [mcMultiClass2Pod2_Heating] -> EnableDifonifmode13/1(mc)
  EnableDifonifmode15/1(enable detection) -> EnableD_H_MultiClass1Pod1_mat/1
  EnableDifonifmode16/1(enable detection) -> EnableD_C_MultiClass1Pod1_mat/1
  [On_Off] -> EnableDifonifmode16/2(on_off), EnableDifonifmode15/2(on_off)
  [mcMultiClass1Pod1_Cooling] -> EnableDifonifmode16/1(mc)
  [mcMultiClass1Pod1_Heating] -> EnableDifonifmode15/1(mc)
  EnableDifonifmode17/1(enable detection) -> EnableD_H_MultiClass2Pod1_mat/1
  EnableDifonifmode18/1(enable detection) -> EnableD_C_MultiClass2Pod1_mat/1
  [On_Off] -> EnableDifonifmode18/2(on_off), EnableDifonifmode17/2(on_off)
  [mcMultiClass2Pod1_Cooling] -> EnableDifonifmode18/1(mc)
  [mcMultiClass2Pod1_Heating] -> EnableDifonifmode17/1(mc)
  EnableDifonifmode19/1(enable detection) -> EnableD_H_MultiClass1Pod2_mat/1
  EnableDifonifmode20/1(enable detection) -> EnableD_C_MultiClass1Pod2_mat/1
  [On_Off] -> EnableDifonifmode20/2(on_off), EnableDifonifmode19/2(on_off)
  [mcMultiClass1Pod2_Cooling] -> EnableDifonifmode20/1(mc)
  [mcMultiClass1Pod2_Heating] -> EnableDifonifmode19/1(mc)
  EnableDifonifmode21/1(enable detection) -> EnableD_H_MultiClass1Pod3_mat/1
  EnableDifonifmode22/1(enable detection) -> EnableD_C_MultiClass1Pod3_mat/1
  [On_Off] -> EnableDifonifmode22/2(on_off), EnableDifonifmode21/2(on_off)
  [mcMultiClass1Pod3_Cooling] -> EnableDifonifmode22/1(mc)
  [mcMultiClass1Pod3_Heating] -> EnableDifonifmode21/1(mc)
  EnableDifonifmode23/1(enable detection) -> EnableD_H_MultiClass2Pod3_mat/1
  EnableDifonifmode24/1(enable detection) -> EnableD_C_MultiClass2Pod3_mat/1
  [On_Off] -> EnableDifonifmode24/2(on_off), EnableDifonifmode23/2(on_off)
  [mcMultiClass2Pod3_Cooling] -> EnableDifonifmode24/1(mc)
  [mcMultiClass2Pod3_Heating] -> EnableDifonifmode23/1(mc)
  EnableDifonifmode25/1(enable detection) -> EnableD_H_CorridorPod1_mat/1
  EnableDifonifmode26/1(enable detection) -> EnableD_C_CorridorPod1_mat/1
  [On_Off] -> EnableDifonifmode26/2(on_off), EnableDifonifmode25/2(on_off)
  [mcCorridorPod1_Cooling] -> EnableDifonifmode26/1(mc)
  [mcCorridorPod1_Heating] -> EnableDifonifmode25/1(mc)
  EnableDifonifmode27/1(enable detection) -> EnableD_H_CorridorPod2_mat/1
  EnableDifonifmode28/1(enable detection) -> EnableD_C_CorridorPod2_mat/1
  [On_Off] -> EnableDifonifmode28/2(on_off), EnableDifonifmode27/2(on_off)
  [mcCorridorPod2_Cooling] -> EnableDifonifmode28/1(mc)
  [mcCorridorPod2_Heating] -> EnableDifonifmode27/1(mc)
  EnableDifonifmode29/1(enable detection) -> EnableD_H_CorridorPod3_mat/1
  EnableDifonifmode30/1(enable detection) -> EnableD_C_CorridorPod3_mat/1
  [On_Off] -> EnableDifonifmode30/2(on_off), EnableDifonifmode29/2(on_off)
  [mcCorridorPod3_Cooling] -> EnableDifonifmode30/1(mc)
  [mcCorridorPod3_Heating] -> EnableDifonifmode29/1(mc)
  EnableDifonifmode31/1(enable detection) -> EnableD_H_MainCorridor_mat/1
  EnableDifonifmode32/1(enable detection) -> EnableD_C_MainCorridor_mat/1
  [On_Off] -> EnableDifonifmode32/2(on_off), EnableDifonifmode31/2(on_off)
  [mcMainCorridor_Cooling] -> EnableDifonifmode32/1(mc)
  [mcMainCorridor_Heating] -> EnableDifonifmode31/1(mc)
  EnableDifonifmode33/1(enable detection) -> EnableD_H_Mech_mat/1
  EnableDifonifmode34/1(enable detection) -> EnableD_C_Mech_mat/1
  [On_Off] -> EnableDifonifmode34/2(on_off), EnableDifonifmode33/2(on_off)
  [mcMech_Cooling] -> EnableDifonifmode34/1(mc)
  [mcMech_Heating] -> EnableDifonifmode33/1(mc)
  EnableDifonifmode35/1(enable detection) -> EnableD_H_ComputerClass_mat/1
  EnableDifonifmode36/1(enable detection) -> EnableD_C_ComputerClass_mat/1
  [On_Off] -> EnableDifonifmode36/2(on_off), EnableDifonifmode35/2(on_off)
  [mcComputerClass_Cooling] -> EnableDifonifmode36/1(mc)
  [mcComputerClass_Heating] -> EnableDifonifmode35/1(mc)
  EnableDifonifmode37/1(enable detection) -> EnableD_H_LibraryMediaCenter_mat/1
  EnableDifonifmode38/1(enable detection) -> EnableD_C_LibraryMediaCenter_mat/1
  [On_Off] -> EnableDifonifmode38/2(on_off), EnableDifonifmode37/2(on_off)
  [mcLibrary_Cooling] -> EnableDifonifmode38/1(mc)
  [mcLibrary_Heating] -> EnableDifonifmode37/1(mc)
  EnableDifonifmode39/1(enable detection) -> EnableD_H_Offices_mat/1
  EnableDifonifmode40/1(enable detection) -> EnableD_C_Offices_mat/1
  [On_Off] -> EnableDifonifmode40/2(on_off), EnableDifonifmode39/2(on_off)
  [mcOffices_Cooling] -> EnableDifonifmode40/1(mc)
  [mcOffices_Heating] -> EnableDifonifmode39/1(mc)
  EnableDifonifmode41/1(enable detection) -> EnableD_H_Lobby_mat/1
  EnableDifonifmode42/1(enable detection) -> EnableD_C_Lobby_mat/1
  [On_Off] -> EnableDifonifmode42/2(on_off), EnableDifonifmode41/2(on_off)
  [mcLobby_Cooling] -> EnableDifonifmode42/1(mc)
  [mcLobby_Heating] -> EnableDifonifmode41/1(mc)
  EnableDifonifmode43/1(enable detection) -> EnableD_H_Cafeteria_mat/1
  EnableDifonifmode44/1(enable detection) -> EnableD_C_Cafeteria_mat/1
  [On_Off] -> EnableDifonifmode44/2(on_off), EnableDifonifmode43/2(on_off)
  [mcCafeteria_Cooling] -> EnableDifonifmode44/1(mc)
  [mcCafeteria_Heating] -> EnableDifonifmode43/1(mc)
  EnableDifonifmode45/1(enable detection) -> EnableD_H_Kitchen_mat/1
  EnableDifonifmode46/1(enable detection) -> EnableD_C_Kitchen_mat/1
  [On_Off] -> EnableDifonifmode46/2(on_off), EnableDifonifmode45/2(on_off)
  [mcKitchen_Cooling] -> EnableDifonifmode46/1(mc)
  [mcKitchen_Heating] -> EnableDifonifmode45/1(mc)
  EnableDifonifmode47/1(enable detection) -> EnableD_H_Gym_mat/1
  EnableDifonifmode48/1(enable detection) -> EnableD_C_Gym_mat/1
  [On_Off] -> EnableDifonifmode48/2(on_off), EnableDifonifmode47/2(on_off)
  [mcGym_Cooling] -> EnableDifonifmode48/1(mc)
  [mcGym_Heating] -> EnableDifonifmode47/1(mc)
  EnableDifonifmode49/1(enable detection) -> EnableD_H_Bath_mat/1
  EnableDifonifmode50/1(enable detection) -> EnableD_C_Bath_mat/1
  [On_Off] -> EnableDifonifmode50/2(on_off), EnableDifonifmode49/2(on_off)
  [mcBath_Cooling] -> EnableDifonifmode50/1(mc)
  [mcBath_Heating] -> EnableDifonifmode49/1(mc)
  Product/1 -> Product1/1, Out1/1, control_inputs_mat/1, Scope/1
  [TcCornerClass1Pod1_Cooling] -> Mux/1
  [TcCornerClass2Pod1_Cooling] -> Mux/2
  [TcCornerClass1Pod2_Cooling] -> Mux/3
  [TcCornerClass2Pod2_Cooling] -> Mux/4
  [TcCornerClass1Pod3_Cooling] -> Mux/5
  [TcCornerClass2Pod3_Cooling] -> Mux/6
  [TcMultiClass1Pod1_Cooling] -> Mux/7
  [TcMultiClass2Pod1_Cooling] -> Mux/8
  [TcMultiClass1Pod2_Cooling] -> Mux/9
  [TcMultiClass2Pod2_Cooling] -> Mux/10
  [TcMultiClass1Pod3_Cooling] -> Mux/11
  [TcMultiClass2Pod3_Cooling] -> Mux/12
  [TcCorridorPod1_Cooling] -> Mux/13
  [TcCorridorPod2_Cooling] -> Mux/14
  [TcCorridorPod3_Cooling] -> Mux/15
  [TcMainCorridor_Cooling] -> Mux/16
  [TcMech_Cooling] -> Mux/17
  [TcComputerClass_Cooling] -> Mux/18
  [TcLibrary_Cooling] -> Mux/19
  [TcOffices_Cooling] -> Mux/20
  [TcLobby_Cooling] -> Mux/21
  [TcCafeteria_Cooling] -> Mux/22
  [TcKitchen_Cooling] -> Mux/23
  [TcGym_Cooling] -> Mux/24
  [TcBath_Cooling] -> Mux/25
  [TcCornerClass1Pod1_Heating] -> Mux53/1
  [TcCornerClass2Pod1_Heating] -> Mux53/2
  [TcCornerClass1Pod2_Heating] -> Mux53/3
  [TcCornerClass2Pod2_Heating] -> Mux53/4
  [TcCornerClass1Pod3_Heating] -> Mux53/5
  [TcCornerClass2Pod3_Heating] -> Mux53/6
  [TcMultiClass1Pod1_Heating] -> Mux53/7
  [TcMultiClass2Pod1_Heating] -> Mux53/8
  [TcMultiClass1Pod2_Heating] -> Mux53/9
  [TcMultiClass2Pod2_Heating] -> Mux53/10
  [TcMultiClass1Pod3_Heating] -> Mux53/11
  [TcMultiClass2Pod3_Heating] -> Mux53/12
  [TcCorridorPod1_Heating] -> Mux53/13
  [TcCorridorPod2_Heating] -> Mux53/14
  [TcCorridorPod3_Heating] -> Mux53/15
  [TcMainCorridor_Heating] -> Mux53/16
  [TcMech_Heating] -> Mux53/17
  [TcComputerClass_Heating] -> Mux53/18
  [TcLibrary_Heating] -> Mux53/19
  [TcOffices_Heating] -> Mux53/20
  [TcLobby_Heating] -> Mux53/21
  [TcCafeteria_Heating] -> Mux53/22
  [TcKitchen_Heating] -> Mux53/23
  [TcGym_Heating] -> Mux53/24
  [TcBath_Heating] -> Mux53/25
  Mux/1 -> Subtract/1
  [Tst_Cooling] -> Subtract/2
  Mux53/1 -> Subtract1/1
  [Tst_Heating] -> Subtract1/2
  Subtract/1 -> Abs1/1
  Subtract1/1 -> Abs/1
  Mux54/1 -> Product1/2
  Abs/1 -> Mux54/2
  Abs1/1 -> Mux54/1
  Product1/1 -> Cpw/1
  Cpw/1 -> EnergyIntegrator/1
  EnergyIntegrator/1 -> Energy/1
  Saturation/1 -> Product/1
  [On_Off] -> Product/2
